simulink model "roach2_sin"  (Simulink 7.9)
settings: Solver=ode45  StartTime=0.0  StopTime=50000  RelTol=1e-3
undefined symbols (not defined in the file or in its folder): sfp
libraries: xbsIndex_r4 x2466, xps_library x13, casper_library_misc x4
(part 2 of 28)

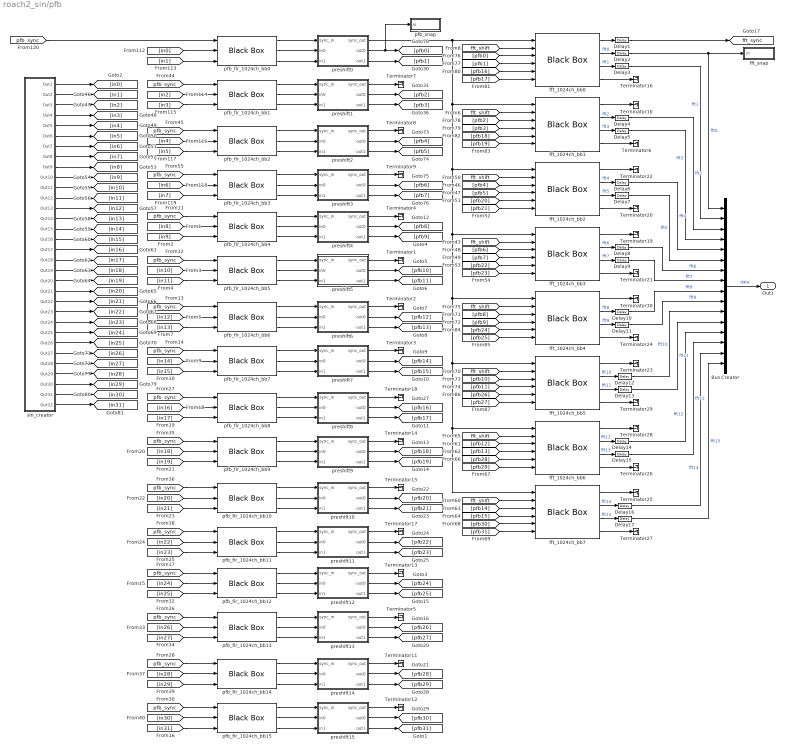
== system roach2_sin/pfb ==
  Bus Creator : BusCreator  Inputs=16
  Delay{1..17} (17 alike) : xbsIndex_r4/Delay  rst=off  infoeditControl=Selection of Reset will increase slice count due to use of real FFs and instead of SRLs  en=off  latency=1  dbl_ovrd=off  reg_retiming=off  block_type=delay
  Terminator{1..30} (30 alike) : Terminator
  fft_1024ch_bb{0..7} (8 alike) : xbsIndex_r4/Black Box  init_code=fft_1024ch_core_config  sim_method=Inactive  verbose=off  block_type=blackbox2
  fft_snap : Subsystem
  pfb_fir_1024ch_bb{0..15} (16 alike) : xbsIndex_r4/Black Box  init_code=pfb_fir_1024ch_core_config  sim_method=Inactive  verbose=off  block_type=blackbox2
  pfb_snap : Subsystem
  preshift0 : Subsystem
  preshift1 : Subsystem
  preshift10 : Subsystem
  preshift11 : Subsystem
  preshift12 : Subsystem
  preshift13 : Subsystem
  preshift14 : Subsystem
  preshift15 : Subsystem
  preshift2 : Subsystem
  preshift3 : Subsystem
  preshift4 : Subsystem
  preshift5 : Subsystem
  preshift6 : Subsystem
  preshift7 : Subsystem
  preshift8 : Subsystem
  preshift9 : Subsystem
  sin_creator : Subsystem
  Out1 : Outport
  tag scope: fft_sync=global
  wires:
  [in0] -> pfb_fir_1024ch_bb0/2
  [in1] -> pfb_fir_1024ch_bb0/3
  [in2] -> pfb_fir_1024ch_bb1/2
  [in3] -> pfb_fir_1024ch_bb1/3
  [in4] -> pfb_fir_1024ch_bb2/2
  [in5] -> pfb_fir_1024ch_bb2/3
  [in6] -> pfb_fir_1024ch_bb3/2
  [in7] -> pfb_fir_1024ch_bb3/3
  pfb_fir_1024ch_bb1/2 -> preshift1/2(in0)
  pfb_fir_1024ch_bb1/3 -> preshift1/3(in1)
  pfb_fir_1024ch_bb2/2 -> preshift2/2(in0)
  pfb_fir_1024ch_bb2/3 -> preshift2/3(in1)
  pfb_fir_1024ch_bb3/2 -> preshift3/2(in0)
  pfb_fir_1024ch_bb3/3 -> preshift3/3(in1)
  [fft_shift] -> fft_1024ch_bb1/2
  fft_1024ch_bb1/4 -> Terminator6/1
  fft_1024ch_bb0/4 -> Terminator16/1
  Delay1/1 -> [fft_sync]
  fft_1024ch_bb1/1 -> Terminator10/1
  [fft_shift] -> fft_1024ch_bb0/2
  [pfb_sync] -> pfb_fir_1024ch_bb0/1
  [pfb_sync] -> pfb_fir_1024ch_bb1/1
  [pfb_sync] -> pfb_fir_1024ch_bb2/1
  [pfb_sync] -> pfb_fir_1024ch_bb3/1
  pfb_fir_1024ch_bb1/1 -> preshift1/1(sync_in)
  pfb_fir_1024ch_bb2/1 -> preshift2/1(sync_in)
  pfb_fir_1024ch_bb3/1 -> preshift3/1(sync_in)
  pfb_fir_1024ch_bb0/1 -> preshift0/1(sync_in)
  pfb_fir_1024ch_bb0/2 -> preshift0/2(in0)
  pfb_fir_1024ch_bb0/3 -> preshift0/3(in1)
  preshift0/1(sync_out) -> fft_1024ch_bb6/1, fft_1024ch_bb7/1, fft_1024ch_bb5/1, fft_1024ch_bb4/1, fft_1024ch_bb3/1, fft_1024ch_bb2/1, fft_1024ch_bb1/1, fft_1024ch_bb0/1
  preshift0/2(out0) -> [pfb0], pfb_snap/1(in)
  preshift0/3(out1) -> [pfb1]
  preshift1/1(sync_out) -> Terminator7/1
  preshift2/1(sync_out) -> Terminator8/1
  preshift3/1(sync_out) -> Terminator9/1
  preshift1/2(out0) -> [pfb2]
  preshift1/3(out1) -> [pfb3]
  preshift2/2(out0) -> [pfb4]
  preshift2/3(out1) -> [pfb5]
  preshift3/2(out0) -> [pfb6]
  preshift3/3(out1) -> [pfb7]
  [pfb0] -> fft_1024ch_bb0/3
  [pfb1] -> fft_1024ch_bb0/4
  [pfb16] -> fft_1024ch_bb0/5
  [pfb17] -> fft_1024ch_bb0/6
  [pfb2] -> fft_1024ch_bb1/3
  [pfb3] -> fft_1024ch_bb1/4
  [pfb18] -> fft_1024ch_bb1/5
  [pfb19] -> fft_1024ch_bb1/6
  [in8] -> pfb_fir_1024ch_bb4/2
  [in9] -> pfb_fir_1024ch_bb4/3
  [in10] -> pfb_fir_1024ch_bb5/2
  [in11] -> pfb_fir_1024ch_bb5/3
  [in12] -> pfb_fir_1024ch_bb6/2
  [in13] -> pfb_fir_1024ch_bb6/3
  [in14] -> pfb_fir_1024ch_bb7/2
  [in15] -> pfb_fir_1024ch_bb7/3
  pfb_fir_1024ch_bb5/2 -> preshift5/2(in0)
  pfb_fir_1024ch_bb5/3 -> preshift5/3(in1)
  pfb_fir_1024ch_bb6/2 -> preshift6/2(in0)
  pfb_fir_1024ch_bb6/3 -> preshift6/3(in1)
  pfb_fir_1024ch_bb7/2 -> preshift7/2(in0)
  pfb_fir_1024ch_bb7/3 -> preshift7/3(in1)
  [pfb_sync] -> pfb_fir_1024ch_bb4/1
  [pfb_sync] -> pfb_fir_1024ch_bb5/1
  [pfb_sync] -> pfb_fir_1024ch_bb6/1
  [pfb_sync] -> pfb_fir_1024ch_bb7/1
  pfb_fir_1024ch_bb5/1 -> preshift5/1(sync_in)
  pfb_fir_1024ch_bb6/1 -> preshift6/1(sync_in)
  pfb_fir_1024ch_bb7/1 -> preshift7/1(sync_in)
  pfb_fir_1024ch_bb4/1 -> preshift4/1(sync_in)
  pfb_fir_1024ch_bb4/2 -> preshift4/2(in0)
  pfb_fir_1024ch_bb4/3 -> preshift4/3(in1)
  preshift4/1(sync_out) -> Terminator4/1
  preshift4/2(out0) -> [pfb8]
  preshift4/3(out1) -> [pfb9]
  preshift5/1(sync_out) -> Terminator1/1
  preshift6/1(sync_out) -> Terminator2/1
  preshift7/1(sync_out) -> Terminator3/1
  preshift5/2(out0) -> [pfb10]
  preshift5/3(out1) -> [pfb11]
  preshift6/2(out0) -> [pfb12]
  preshift6/3(out1) -> [pfb13]
  preshift7/2(out0) -> [pfb14]
  preshift7/3(out1) -> [pfb15]
  [in16] -> pfb_fir_1024ch_bb8/2
  [in17] -> pfb_fir_1024ch_bb8/3
  [in18] -> pfb_fir_1024ch_bb9/2
  [in19] -> pfb_fir_1024ch_bb9/3
  [in20] -> pfb_fir_1024ch_bb10/2
  [in21] -> pfb_fir_1024ch_bb10/3
  [in22] -> pfb_fir_1024ch_bb11/2
  [in23] -> pfb_fir_1024ch_bb11/3
  pfb_fir_1024ch_bb9/2 -> preshift9/2(in0)
  pfb_fir_1024ch_bb9/3 -> preshift9/3(in1)
  pfb_fir_1024ch_bb10/2 -> preshift10/2(in0)
  pfb_fir_1024ch_bb10/3 -> preshift10/3(in1)
  pfb_fir_1024ch_bb11/2 -> preshift11/2(in0)
  pfb_fir_1024ch_bb11/3 -> preshift11/3(in1)
  [pfb_sync] -> pfb_fir_1024ch_bb8/1
  [pfb_sync] -> pfb_fir_1024ch_bb9/1
  [pfb_sync] -> pfb_fir_1024ch_bb10/1
  [pfb_sync] -> pfb_fir_1024ch_bb11/1
  pfb_fir_1024ch_bb9/1 -> preshift9/1(sync_in)
  pfb_fir_1024ch_bb10/1 -> preshift10/1(sync_in)
  pfb_fir_1024ch_bb11/1 -> preshift11/1(sync_in)
  pfb_fir_1024ch_bb8/1 -> preshift8/1(sync_in)
  pfb_fir_1024ch_bb8/2 -> preshift8/2(in0)
  pfb_fir_1024ch_bb8/3 -> preshift8/3(in1)
  preshift8/1(sync_out) -> Terminator18/1
  preshift8/2(out0) -> [pfb16]
  preshift8/3(out1) -> [pfb17]
  preshift9/1(sync_out) -> Terminator14/1
  preshift10/1(sync_out) -> Terminator15/1
  preshift11/1(sync_out) -> Terminator17/1
  preshift9/2(out0) -> [pfb18]
  preshift9/3(out1) -> [pfb19]
  preshift10/2(out0) -> [pfb20]
  preshift10/3(out1) -> [pfb21]
  preshift11/2(out0) -> [pfb22]
  preshift11/3(out1) -> [pfb23]
  [in24] -> pfb_fir_1024ch_bb12/2
  [in25] -> pfb_fir_1024ch_bb12/3
  [in26] -> pfb_fir_1024ch_bb13/2
  [in27] -> pfb_fir_1024ch_bb13/3
  [in28] -> pfb_fir_1024ch_bb14/2
  [in29] -> pfb_fir_1024ch_bb14/3
  [in30] -> pfb_fir_1024ch_bb15/2
  [in31] -> pfb_fir_1024ch_bb15/3
  pfb_fir_1024ch_bb13/2 -> preshift13/2(in0)
  pfb_fir_1024ch_bb13/3 -> preshift13/3(in1)
  pfb_fir_1024ch_bb14/2 -> preshift14/2(in0)
  pfb_fir_1024ch_bb14/3 -> preshift14/3(in1)
  pfb_fir_1024ch_bb15/2 -> preshift15/2(in0)
  pfb_fir_1024ch_bb15/3 -> preshift15/3(in1)
  [pfb_sync] -> pfb_fir_1024ch_bb12/1
  [pfb_sync] -> pfb_fir_1024ch_bb13/1
  [pfb_sync] -> pfb_fir_1024ch_bb14/1
  [pfb_sync] -> pfb_fir_1024ch_bb15/1
  pfb_fir_1024ch_bb13/1 -> preshift13/1(sync_in)
  pfb_fir_1024ch_bb14/1 -> preshift14/1(sync_in)
  pfb_fir_1024ch_bb15/1 -> preshift15/1(sync_in)
  pfb_fir_1024ch_bb12/1 -> preshift12/1(sync_in)
  pfb_fir_1024ch_bb12/2 -> preshift12/2(in0)
  pfb_fir_1024ch_bb12/3 -> preshift12/3(in1)
  preshift12/1(sync_out) -> Terminator13/1
  preshift12/2(out0) -> [pfb24]
  preshift12/3(out1) -> [pfb25]
  preshift13/1(sync_out) -> Terminator5/1
  preshift14/1(sync_out) -> Terminator11/1
  preshift15/1(sync_out) -> Terminator12/1
  preshift13/2(out0) -> [pfb26]
  preshift13/3(out1) -> [pfb27]
  preshift14/2(out0) -> [pfb28]
  preshift14/3(out1) -> [pfb29]
  preshift15/2(out0) -> [pfb30]
  preshift15/3(out1) -> [pfb31]
  [fft_shift] -> fft_1024ch_bb3/2
  fft_1024ch_bb3/4 -> Terminator21/1
  fft_1024ch_bb2/4 -> Terminator20/1
  fft_1024ch_bb2/1 -> Terminator22/1
  fft_1024ch_bb3/1 -> Terminator19/1
  [fft_shift] -> fft_1024ch_bb2/2
  [pfb4] -> fft_1024ch_bb2/3
  [pfb5] -> fft_1024ch_bb2/4
  [pfb20] -> fft_1024ch_bb2/5
  [pfb21] -> fft_1024ch_bb2/6
  [pfb6] -> fft_1024ch_bb3/3
  [pfb7] -> fft_1024ch_bb3/4
  [pfb22] -> fft_1024ch_bb3/5
  [pfb23] -> fft_1024ch_bb3/6
  [fft_shift] -> fft_1024ch_bb5/2
  fft_1024ch_bb5/4 -> Terminator29/1
  fft_1024ch_bb4/4 -> Terminator24/1
  fft_1024ch_bb4/1 -> Terminator30/1
  fft_1024ch_bb5/1 -> Terminator23/1
  [fft_shift] -> fft_1024ch_bb4/2
  [pfb8] -> fft_1024ch_bb4/3
  [pfb9] -> fft_1024ch_bb4/4
  [pfb24] -> fft_1024ch_bb4/5
  [pfb25] -> fft_1024ch_bb4/6
  [pfb10] -> fft_1024ch_bb5/3
  [pfb11] -> fft_1024ch_bb5/4
  [pfb26] -> fft_1024ch_bb5/5
  [pfb27] -> fft_1024ch_bb5/6
  [fft_shift] -> fft_1024ch_bb7/2
  fft_1024ch_bb7/4 -> Terminator27/1
  fft_1024ch_bb6/4 -> Terminator26/1
  fft_1024ch_bb6/1 -> Terminator28/1
  fft_1024ch_bb7/1 -> Terminator25/1
  [fft_shift] -> fft_1024ch_bb6/2
  [pfb12] -> fft_1024ch_bb6/3
  [pfb13] -> fft_1024ch_bb6/4
  [pfb28] -> fft_1024ch_bb6/5
  [pfb29] -> fft_1024ch_bb6/6
  [pfb14] -> fft_1024ch_bb7/3
  [pfb15] -> fft_1024ch_bb7/4
  [pfb30] -> fft_1024ch_bb7/5
  [pfb31] -> fft_1024ch_bb7/6
  Delay2/1 -> Bus Creator/1, fft_snap/1(in)  "fft0"
  Delay3/1 -> Bus Creator/2  "fft1"
  Delay4/1 -> Bus Creator/3  "fft2"
  Delay5/1 -> Bus Creator/4  "fft3"
  Delay6/1 -> Bus Creator/5  "fft4"
  Delay7/1 -> Bus Creator/6  "fft5"
  Delay8/1 -> Bus Creator/7  "fft6"
  Delay9/1 -> Bus Creator/8  "fft7"
  Delay10/1 -> Bus Creator/9  "fft8"
  Delay11/1 -> Bus Creator/10  "fft9"
  Delay12/1 -> Bus Creator/11  "fft10"
  Delay13/1 -> Bus Creator/12  "fft11"
  Delay14/1 -> Bus Creator/13  "fft12"
  Delay15/1 -> Bus Creator/14  "fft13"
  Delay16/1 -> Bus Creator/15  "fft14"
  Delay17/1 -> Bus Creator/16  "fft15"
  Bus Creator/1 -> Out1/1  "data"
  fft_1024ch_bb7/2 -> Delay16/1  "fft14"
  fft_1024ch_bb7/3 -> Delay17/1  "fft15"
  fft_1024ch_bb6/2 -> Delay14/1  "fft12"
  fft_1024ch_bb6/3 -> Delay15/1  "fft13"
  fft_1024ch_bb5/2 -> Delay12/1  "fft10"
  fft_1024ch_bb5/3 -> Delay13/1  "fft11"
  fft_1024ch_bb0/2 -> Delay2/1  "fft0"
  fft_1024ch_bb0/1 -> Delay1/1
  fft_1024ch_bb0/3 -> Delay3/1  "fft1"
  fft_1024ch_bb1/2 -> Delay4/1  "fft2"
  fft_1024ch_bb1/3 -> Delay5/1  "fft3"
  fft_1024ch_bb2/2 -> Delay6/1  "fft4"
  fft_1024ch_bb2/3 -> Delay7/1  "fft5"
  fft_1024ch_bb3/2 -> Delay8/1  "fft6"
  fft_1024ch_bb3/3 -> Delay9/1  "fft7"
  fft_1024ch_bb4/2 -> Delay10/1  "fft8"
  fft_1024ch_bb4/3 -> Delay11/1  "fft9"
  sin_creator/1(Out1) -> [in0]
  sin_creator/2(Out2) -> [in1]
  sin_creator/3(Out3) -> [in2]
  sin_creator/4(Out4) -> [in3]
  sin_creator/5(Out5) -> [in4]
  sin_creator/6(Out6) -> [in5]
  sin_creator/7(Out7) -> [in6]
  sin_creator/8(Out8) -> [in7]
  sin_creator/9(Out9) -> [in8]
  sin_creator/10(Out10) -> [in9]
  sin_creator/11(Out11) -> [in10]
  sin_creator/12(Out12) -> [in11]
  sin_creator/13(Out13) -> [in12]
  sin_creator/14(Out14) -> [in13]
  sin_creator/15(Out15) -> [in14]
  sin_creator/16(Out16) -> [in15]
  sin_creator/17(Out17) -> [in16]
  sin_creator/18(Out18) -> [in17]
  sin_creator/19(Out19) -> [in18]
  sin_creator/20(Out20) -> [in19]
  sin_creator/21(Out21) -> [in20]
  sin_creator/22(Out22) -> [in21]
  sin_creator/23(Out23) -> [in22]
  sin_creator/24(Out24) -> [in23]
  sin_creator/25(Out25) -> [in24]
  sin_creator/26(Out26) -> [in25]
  sin_creator/27(Out27) -> [in26]
  sin_creator/28(Out28) -> [in27]
  sin_creator/29(Out29) -> [in28]
  sin_creator/30(Out30) -> [in29]
  sin_creator/31(Out31) -> [in30]
  sin_creator/32(Out32) -> [in31]

== system roach2_sin/rcs ==
  note: 0 -> revision info
      | 1 -> timestamp
  note: Last modification
      | in seconds since
      | 01-01-1970
  note: 0 -> git
      | 1 -> svn
  note: 0 -> cleanly in revision control
  note: git -> first 28 bits of commit hash
      | svn -> latest revision number
  note: 0 -> revision info
      | 1 -> timestamp
  note: Compile time
      | in seconds since
      | 01-01-1970
  note: 0 -> git
      | 1 -> svn
  note: 0 -> cleanly in revision control
  note: git -> first 28 bits of commit hash
      | svn -> latest revision number
  Concat : xbsIndex_r4/Concat  num_inputs=2  dbl_ovrd=off  block_type=concat
  Concat1 : xbsIndex_r4/Concat  num_inputs=2  dbl_ovrd=off  block_type=concat
  Mux : xbsIndex_r4/Mux  inputs=2  en=off  latency=0  precision=Full  arith_type=Unsigned  n_bits=16  bin_pt=14  quantization=Truncate  overflow=Wrap  dbl_ovrd=off  block_type=mux
      settings: block_version=11.4
  Mux1 : xbsIndex_r4/Mux  inputs=2  en=off  latency=0  precision=Full  arith_type=Unsigned  n_bits=16  bin_pt=14  quantization=Truncate  overflow=Wrap  dbl_ovrd=off  block_type=mux
      settings: block_version=11.4
  Terminator : Terminator
  Terminator1 : Terminator
  Terminator2 : Terminator
  app : Subsystem  Tag=xps:sw_reg  mask{io_dir=To Processor, arith_type=Unsigned, bitwidth=32, bin_pt=0, sample_period=1, latency=0}
      mask prompts: io_dir "I/O direction"; arith_type "Data Type"; bitwidth "Data bitwidth"; bin_pt "Data binary point"; sample_period "Sample period"
      mask init:
      | gw_name = clear_name(gcb);
      | config.source = str2func('software_register_init');
      | config.toplevel = gcb;
      | xBlock( config, {io_dir, arith_type, bitwidth, bin_pt, ... 
      |     sample_period, gw_name, latency});
      mask StyleString: popup(From Processor|To Processor),popup(Unsigned|Signed  (2's comp)),edit,edit,edit,edit
  app_dirty : xbsIndex_r4/Constant  const=1  gui_display_data_type=Fixed-point  arith_type=Unsigned  n_bits=1  bin_pt=0  preci_type=Single  exp_width=8  frac_width=24  explicit_period=on  period=1  equ=P=C  opselect=C  inp2=PCIN>>17  opr=+  inp1=P  carry=CIN  dbl_ovrd=off  block_type=constant
      settings: block_version=11.4
  app_info : xbsIndex_r4/Concat  num_inputs=4  dbl_ovrd=off  block_type=concat
  app_info1 : xbsIndex_r4/Concat  num_inputs=4  dbl_ovrd=off  block_type=concat
  app_rcs_type : xbsIndex_r4/Constant  const=0  gui_display_data_type=Fixed-point  arith_type=Unsigned  n_bits=1  bin_pt=0  preci_type=Single  exp_width=8  frac_width=24  explicit_period=on  period=1  equ=P=C  opselect=C  inp2=PCIN>>17  opr=+  inp1=P  carry=CIN  dbl_ovrd=off  block_type=constant
      settings: block_version=11.4
  app_reserved : xbsIndex_r4/Constant  const=0  gui_display_data_type=Fixed-point  arith_type=Unsigned  n_bits=1  bin_pt=0  preci_type=Single  exp_width=8  frac_width=24  explicit_period=on  period=1  equ=P=C  opselect=C  inp2=PCIN>>17  opr=+  inp1=P  carry=CIN  dbl_ovrd=off  block_type=constant
      settings: block_version=11.4
  app_revision : xbsIndex_r4/Constant  const=0  gui_display_data_type=Fixed-point  arith_type=Unsigned  n_bits=28  bin_pt=0  preci_type=Single  exp_width=8  frac_width=24  explicit_period=on  period=1  equ=P=C  opselect=C  inp2=PCIN>>17  opr=+  inp1=P  carry=CIN  dbl_ovrd=off  block_type=constant
      settings: block_version=11.4
  app_timestamp : xbsIndex_r4/Constant  const=1445445588  gui_display_data_type=Fixed-point  arith_type=Unsigned  n_bits=31  bin_pt=0  preci_type=Single  exp_width=8  frac_width=24  explicit_period=on  period=1  equ=P=C  opselect=C  inp2=PCIN>>17  opr=+  inp1=P  carry=CIN  dbl_ovrd=off  block_type=constant
      settings: block_version=11.4
  app_type : xbsIndex_r4/Constant  const=1  gui_display_data_type=Fixed-point  arith_type=Unsigned  n_bits=1  bin_pt=0  preci_type=Single  exp_width=8  frac_width=24  explicit_period=on  period=1  equ=P=C  opselect=C  inp2=PCIN>>17  opr=+  inp1=P  carry=CIN  dbl_ovrd=off  block_type=constant
      settings: block_version=11.4
  lib : Subsystem  Tag=xps:sw_reg  mask{io_dir=To Processor, arith_type=Unsigned, bitwidth=32, bin_pt=0, sample_period=1, latency=0}
      mask prompts: io_dir "I/O direction"; arith_type "Data Type"; bitwidth "Data bitwidth"; bin_pt "Data binary point"; sample_period "Sample period"
      mask init:
      | gw_name = clear_name(gcb);
      | config.source = str2func('software_register_init');
      | config.toplevel = gcb;
      | xBlock( config, {io_dir, arith_type, bitwidth, bin_pt, ... 
      |     sample_period, gw_name, latency});
      mask StyleString: popup(From Processor|To Processor),popup(Unsigned|Signed  (2's comp)),edit,edit,edit,edit
  lib_dirty : xbsIndex_r4/Constant  const=1  gui_display_data_type=Fixed-point  arith_type=Unsigned  n_bits=1  bin_pt=0  preci_type=Single  exp_width=8  frac_width=24  explicit_period=on  period=1  equ=P=C  opselect=C  inp2=PCIN>>17  opr=+  inp1=P  carry=CIN  dbl_ovrd=off  block_type=constant
      settings: block_version=11.4
  lib_rcs_type : xbsIndex_r4/Constant  const=0  gui_display_data_type=Fixed-point  arith_type=Unsigned  n_bits=1  bin_pt=0  preci_type=Single  exp_width=8  frac_width=24  explicit_period=on  period=1  equ=P=C  opselect=C  inp2=PCIN>>17  opr=+  inp1=P  carry=CIN  dbl_ovrd=off  block_type=constant
      settings: block_version=11.4
  lib_reserved : xbsIndex_r4/Constant  const=0  gui_display_data_type=Fixed-point  arith_type=Unsigned  n_bits=1  bin_pt=0  preci_type=Single  exp_width=8  frac_width=24  explicit_period=on  period=1  equ=P=C  opselect=C  inp2=PCIN>>17  opr=+  inp1=P  carry=CIN  dbl_ovrd=off  block_type=constant
      settings: block_version=11.4
  lib_revision : xbsIndex_r4/Constant  const=hex2dec('2f40dad')  gui_display_data_type=Fixed-point  arith_type=Unsigned  n_bits=28  bin_pt=0  preci_type=Single  exp_width=8  frac_width=24  explicit_period=on  period=1  equ=P=C  opselect=C  inp2=PCIN>>17  opr=+  inp1=P  carry=CIN  dbl_ovrd=off  block_type=constant
      settings: block_version=11.4
  lib_timestamp : xbsIndex_r4/Constant  const=1445474578  gui_display_data_type=Fixed-point  arith_type=Unsigned  n_bits=31  bin_pt=0  preci_type=Single  exp_width=8  frac_width=24  explicit_period=on  period=1  equ=P=C  opselect=C  inp2=PCIN>>17  opr=+  inp1=P  carry=CIN  dbl_ovrd=off  block_type=constant
      settings: block_version=11.4
  lib_type : xbsIndex_r4/Constant  const=0  gui_display_data_type=Fixed-point  arith_type=Unsigned  n_bits=1  bin_pt=0  preci_type=Single  exp_width=8  frac_width=24  explicit_period=on  period=1  equ=P=C  opselect=C  inp2=PCIN>>17  opr=+  inp1=P  carry=CIN  dbl_ovrd=off  block_type=constant
      settings: block_version=11.4
  user : Subsystem  Tag=xps:sw_reg  mask{io_dir=To Processor, arith_type=Unsigned, bitwidth=32, bin_pt=0, sample_period=1, latency=0}
      mask prompts: io_dir "I/O direction"; arith_type "Data Type"; bitwidth "Data bitwidth"; bin_pt "Data binary point"; sample_period "Sample period"
      mask init:
      | gw_name = clear_name(gcb);
      | config.source = str2func('software_register_init');
      | config.toplevel = gcb;
      | xBlock( config, {io_dir, arith_type, bitwidth, bin_pt, ... 
      |     sample_period, gw_name, latency});
      mask StyleString: popup(From Processor|To Processor),popup(Unsigned|Signed  (2's comp)),edit,edit,edit,edit
  user_val : xbsIndex_r4/Constant  const=user_val  gui_display_data_type=Fixed-point  arith_type=Unsigned  n_bits=32  bin_pt=0  preci_type=Single  exp_width=8  frac_width=24  explicit_period=off  period=1  equ=P=C  opselect=C  inp2=PCIN>>17  opr=+  inp1=P  carry=CIN  dbl_ovrd=off  block_type=constant
      settings: block_version=11.4
  wires:
  app/1(sim_reg_out) -> Terminator/1
  user/1(sim_reg_out) -> Terminator2/1
  user_val/1 -> user/1(reg_out)
  app_rcs_type/1 -> app_info/1
  app_reserved/1 -> app_info/2
  app_dirty/1 -> app_info/3
  app_revision/1 -> app_info/4
  app_type/1 -> Concat/1, Mux/1
  app_info/1 -> Mux/2
  app_timestamp/1 -> Mux/3
  Mux/1 -> Concat/2
  Concat/1 -> app/1(reg_out)
  lib/1(sim_reg_out) -> Terminator1/1
  lib_rcs_type/1 -> app_info1/1
  lib_reserved/1 -> app_info1/2
  lib_dirty/1 -> app_info1/3
  lib_revision/1 -> app_info1/4
  lib_type/1 -> Concat1/1, Mux1/1
  app_info1/1 -> Mux1/2
  lib_timestamp/1 -> Mux1/3
  Mux1/1 -> Concat1/2
  Concat1/1 -> lib/1(reg_out)

== system roach2_sin/seed ==
  0 : Subsystem  Tag=xps:sw_reg  AncestorBlock=xps_library/software register  mask{numios=1, io_dir=From Processor, io_delay=0, sample_period=1, name1=reg, bitwidth1=32, arith_type1=Unsigned, bin_pt1=0}
      mask prompts: numios "Num I/Os"; io_dir "I/O direction"; io_delay "I/O delay"; sample_period "Sample period"; name1 "Name"; bitwidth1 "Bitwidth"; arith_type1 "Type"; bin_pt1 "Binary pt"
      mask init:
      | swreg_init(gcb);
      mask StyleString: edit,popup(To Processor|From Processor),edit,edit,edit,edit,popup(Unsigned|Signed  (2's comp)|Boolean),edit
      mask CallbackString:
      | t = timer('StartDelay', 0.1); t.TimerFcn = {@swreg_cb, gcb}; start(t);|a = get_param(gcb, 'MaskEnables');
      | if strcmp(get_param(gcb, 'io_dir'), 'To Processor'),
      | a(4) = {'off'};
      | else
      | a(4) = {'on'};
      | end
      | set_param(gcb, 'MaskEnables', a);
      | |||||a = get_param(gcb, 'MaskEnables');
      | if strcmp(get_param(gcb, 'arith_type1'), 'Boolean'),
      | set_param(gcb, 'bitwidth1', '1');
      | set_param(gcb, 'bin_pt1', '0');
      | a(6) = {'off'};
      | a(8) = {'off'};
      | else
      | a(6) = {'on'};
      | a(8) = {'on'};
      | end
      | set_param(gcb, 'MaskEnables', a);
      | |
      mask TabNameString: Setup,Setup,Setup,Setup,IO1,IO1,IO1,IO1
  1 : Subsystem  Tag=xps:sw_reg  AncestorBlock=xps_library/software register  mask{numios=1, io_dir=From Processor, io_delay=0, sample_period=1, name1=reg, bitwidth1=32, arith_type1=Unsigned, bin_pt1=0}
      mask prompts: numios "Num I/Os"; io_dir "I/O direction"; io_delay "I/O delay"; sample_period "Sample period"; name1 "Name"; bitwidth1 "Bitwidth"; arith_type1 "Type"; bin_pt1 "Binary pt"
      mask init:
      | swreg_init(gcb);
      mask StyleString: edit,popup(To Processor|From Processor),edit,edit,edit,edit,popup(Unsigned|Signed  (2's comp)|Boolean),edit
      mask CallbackString:
      | t = timer('StartDelay', 0.1); t.TimerFcn = {@swreg_cb, gcb}; start(t);|a = get_param(gcb, 'MaskEnables');
      | if strcmp(get_param(gcb, 'io_dir'), 'To Processor'),
      | a(4) = {'off'};
      | else
      | a(4) = {'on'};
      | end
      | set_param(gcb, 'MaskEnables', a);
      | |||||a = get_param(gcb, 'MaskEnables');
      | if strcmp(get_param(gcb, 'arith_type1'), 'Boolean'),
      | set_param(gcb, 'bitwidth1', '1');
      | set_param(gcb, 'bin_pt1', '0');
      | a(6) = {'off'};
      | a(8) = {'off'};
      | else
      | a(6) = {'on'};
      | a(8) = {'on'};
      | end
      | set_param(gcb, 'MaskEnables', a);
      | |
      mask TabNameString: Setup,Setup,Setup,Setup,IO1,IO1,IO1,IO1
  2 : Subsystem  Tag=xps:sw_reg  AncestorBlock=xps_library/software register  mask{numios=1, io_dir=From Processor, io_delay=0, sample_period=1, name1=reg, bitwidth1=32, arith_type1=Unsigned, bin_pt1=0}
      mask prompts: numios "Num I/Os"; io_dir "I/O direction"; io_delay "I/O delay"; sample_period "Sample period"; name1 "Name"; bitwidth1 "Bitwidth"; arith_type1 "Type"; bin_pt1 "Binary pt"
      mask init:
      | swreg_init(gcb);
      mask StyleString: edit,popup(To Processor|From Processor),edit,edit,edit,edit,popup(Unsigned|Signed  (2's comp)|Boolean),edit
      mask CallbackString:
      | t = timer('StartDelay', 0.1); t.TimerFcn = {@swreg_cb, gcb}; start(t);|a = get_param(gcb, 'MaskEnables');
      | if strcmp(get_param(gcb, 'io_dir'), 'To Processor'),
      | a(4) = {'off'};
      | else
      | a(4) = {'on'};
      | end
      | set_param(gcb, 'MaskEnables', a);
      | |||||a = get_param(gcb, 'MaskEnables');
      | if strcmp(get_param(gcb, 'arith_type1'), 'Boolean'),
      | set_param(gcb, 'bitwidth1', '1');
      | set_param(gcb, 'bin_pt1', '0');
      | a(6) = {'off'};
      | a(8) = {'off'};
      | else
      | a(6) = {'on'};
      | a(8) = {'on'};
      | end
      | set_param(gcb, 'MaskEnables', a);
      | |
      mask TabNameString: Setup,Setup,Setup,Setup,IO1,IO1,IO1,IO1
  3 : Subsystem  Tag=xps:sw_reg  AncestorBlock=xps_library/software register  mask{numios=1, io_dir=From Processor, io_delay=0, sample_period=1, name1=reg, bitwidth1=32, arith_type1=Unsigned, bin_pt1=0}
      mask prompts: numios "Num I/Os"; io_dir "I/O direction"; io_delay "I/O delay"; sample_period "Sample period"; name1 "Name"; bitwidth1 "Bitwidth"; arith_type1 "Type"; bin_pt1 "Binary pt"
      mask init:
      | swreg_init(gcb);
      mask StyleString: edit,popup(To Processor|From Processor),edit,edit,edit,edit,popup(Unsigned|Signed  (2's comp)|Boolean),edit
      mask CallbackString:
      | t = timer('StartDelay', 0.1); t.TimerFcn = {@swreg_cb, gcb}; start(t);|a = get_param(gcb, 'MaskEnables');
      | if strcmp(get_param(gcb, 'io_dir'), 'To Processor'),
      | a(4) = {'off'};
      | else
      | a(4) = {'on'};
      | end
      | set_param(gcb, 'MaskEnables', a);
      | |||||a = get_param(gcb, 'MaskEnables');
      | if strcmp(get_param(gcb, 'arith_type1'), 'Boolean'),
      | set_param(gcb, 'bitwidth1', '1');
      | set_param(gcb, 'bin_pt1', '0');
      | a(6) = {'off'};
      | a(8) = {'off'};
      | else
      | a(6) = {'on'};
      | a(8) = {'on'};
      | end
      | set_param(gcb, 'MaskEnables', a);
      | |
      mask TabNameString: Setup,Setup,Setup,Setup,IO1,IO1,IO1,IO1
  BitBasher : xbsIndex_r4/BitBasher  bitexpr=|  display_expr=off  sr_1=1  arith_type1=Unsigned  bin_pt1=0  sr_2=2  arith_type2=Unsigned  bin_pt2=0  sr_3=3  arith_type3=Unsigned  bin_pt3=0  sr_4=4  arith_type4=Unsigned  bin_pt4=0  dbl_ovrd=off  block_type=bitbasher
      | <!DOCTYPE HTML PUBLIC "-//W3C//DTD HTML 4.0//EN" "http://www.w3.org/TR/REC-html40/strict.dtd">
      | <html><head><meta name="qrichtext" content="1" /><style type="text/css">
      | p, li { white-space: pre-wrap; }
      | </style></head><body style=" font-family:'Sans'; font-size:10pt; font-weight:400; font-style:normal;">
      | <p style=" margin-top:12px; margin-bottom:12px; margin-left:0px; margin-right:0px; -qt-block-indent:0; text-indent:0px;"><span style=" font-family:'MS Shell Dlg'; font-size:8pt;">s0={8'h5c, s32[7:0], s32[0:7], 8'ha3}<br />s1={8'h5c, s32[15:8], s32[8:15], 8'ha3}<br />s2={8'h5c, s32[23:16], s32[16:23], 8'ha3}<br />s3={8'h5c, s32[31:24], s32[24:31], 8'ha3}</span></p></body></html>
  BitBasher1 : xbsIndex_r4/BitBasher  bitexpr=|  display_expr=off  sr_1=1  arith_type1=Unsigned  bin_pt1=0  sr_2=2  arith_type2=Unsigned  bin_pt2=0  sr_3=3  arith_type3=Unsigned  bin_pt3=0  sr_4=4  arith_type4=Unsigned  bin_pt4=0  dbl_ovrd=off  block_type=bitbasher
      | <!DOCTYPE HTML PUBLIC "-//W3C//DTD HTML 4.0//EN" "http://www.w3.org/TR/REC-html40/strict.dtd">
      | <html><head><meta name="qrichtext" content="1" /><style type="text/css">
      | p, li { white-space: pre-wrap; }
      | </style></head><body style=" font-family:'Sans'; font-size:10pt; font-weight:400; font-style:normal;">
      | <p style=" margin-top:12px; margin-bottom:12px; margin-left:0px; margin-right:0px; -qt-block-indent:0; text-indent:0px;"><span style=" font-family:'MS Shell Dlg'; font-size:8pt;">s0={8'h5c, s32[7:0], s32[0:7], 8'ha3}<br />s1={8'h5c, s32[15:8], s32[8:15], 8'ha3}<br />s2={8'h5c, s32[23:16], s32[16:23], 8'ha3}<br />s3={8'h5c, s32[31:24], s32[24:31], 8'ha3}</span></p></body></html>
  BitBasher2 : xbsIndex_r4/BitBasher  bitexpr=|  display_expr=off  sr_1=1  arith_type1=Unsigned  bin_pt1=0  sr_2=2  arith_type2=Unsigned  bin_pt2=0  sr_3=3  arith_type3=Unsigned  bin_pt3=0  sr_4=4  arith_type4=Unsigned  bin_pt4=0  dbl_ovrd=off  block_type=bitbasher
      | <!DOCTYPE HTML PUBLIC "-//W3C//DTD HTML 4.0//EN" "http://www.w3.org/TR/REC-html40/strict.dtd">
      | <html><head><meta name="qrichtext" content="1" /><style type="text/css">
      | p, li { white-space: pre-wrap; }
      | </style></head><body style=" font-family:'Sans'; font-size:10pt; font-weight:400; font-style:normal;">
      | <p style=" margin-top:12px; margin-bottom:12px; margin-left:0px; margin-right:0px; -qt-block-indent:0; text-indent:0px;"><span style=" font-family:'MS Shell Dlg'; font-size:8pt;">s0={8'h5c, s32[7:0], s32[0:7], 8'ha3}<br />s1={8'h5c, s32[15:8], s32[8:15], 8'ha3}<br />s2={8'h5c, s32[23:16], s32[16:23], 8'ha3}<br />s3={8'h5c, s32[31:24], s32[24:31], 8'ha3}</span></p></body></html>
  BitBasher3 : xbsIndex_r4/BitBasher  bitexpr=|  display_expr=off  sr_1=1  arith_type1=Unsigned  bin_pt1=0  sr_2=2  arith_type2=Unsigned  bin_pt2=0  sr_3=3  arith_type3=Unsigned  bin_pt3=0  sr_4=4  arith_type4=Unsigned  bin_pt4=0  dbl_ovrd=off  block_type=bitbasher
      | <!DOCTYPE HTML PUBLIC "-//W3C//DTD HTML 4.0//EN" "http://www.w3.org/TR/REC-html40/strict.dtd">
      | <html><head><meta name="qrichtext" content="1" /><style type="text/css">
      | p, li { white-space: pre-wrap; }
      | </style></head><body style=" font-family:'Sans'; font-size:10pt; font-weight:400; font-style:normal;">
      | <p style=" margin-top:12px; margin-bottom:12px; margin-left:0px; margin-right:0px; -qt-block-indent:0; text-indent:0px;"><span style=" font-family:'MS Shell Dlg'; font-size:8pt;">s0={8'h5c, s32[7:0], s32[0:7], 8'ha3}<br />s1={8'h5c, s32[15:8], s32[8:15], 8'ha3}<br />s2={8'h5c, s32[23:16], s32[16:23], 8'ha3}<br />s3={8'h5c, s32[31:24], s32[24:31], 8'ha3}</span></p></body></html>
  Constant{,1..3} (4 alike) : Constant  Value=0
  armed_trigger : casper_library_misc/armed_trigger
  tag scope: seed0=global, seed10=global, seed11=global, seed12=global, seed13=global, seed14=global, seed15=global, seed1=global, seed2=global, seed3=global, seed4=global, seed5=global, seed6=global, seed7=global, seed8=global, seed9=global, seed_ld=global
  wires:
  Constant3/1 -> 3/1(sim_reg)
  Constant2/1 -> 2/1(sim_reg)
  Constant1/1 -> 1/1(sim_reg)
  1/1(in_reg) -> BitBasher1/1
  Constant/1 -> 0/1(sim_reg)
  0/1(in_reg) -> BitBasher/1
  BitBasher/1 -> [seed0]
  BitBasher/2 -> [seed1]
  BitBasher/3 -> [seed2]
  BitBasher/4 -> [seed3]
  BitBasher1/1 -> [seed4]
  BitBasher1/2 -> [seed5]
  BitBasher1/3 -> [seed6]
  BitBasher1/4 -> [seed7]
  BitBasher2/1 -> [seed8]
  BitBasher2/2 -> [seed9]
  BitBasher2/3 -> [seed10]
  BitBasher2/4 -> [seed11]
  BitBasher3/1 -> [seed12]
  BitBasher3/2 -> [seed13]
  BitBasher3/3 -> [seed14]
  BitBasher3/4 -> [seed15]
  3/1(in_reg) -> BitBasher3/1
  2/1(in_reg) -> BitBasher2/1
  [arm_noise] -> armed_trigger/1
  [sync_in] -> armed_trigger/2
  armed_trigger/1 -> [seed_ld]

== system roach2_sin/snap ==
  0 : Subsystem  Tag=casper:snapshot  AncestorBlock=casper_library_scopes/snapshot  mask(snapshot){storage=bram, dram_dimm=1, dram_clock=200, nsamples=12, data_width=32, offset=off, circap=off, value=off, use_dsp48=off}
      mask prompts: storage "Storage medium"; dram_dimm "DRAM dimm"; dram_clock "DRAM clock rate"; nsamples "Number of Samples (2^?)"; data_width "Data width"; offset "Start delay support"; circap "Circular capture support"; value "Extra value capture support"; use_dsp48 "Use DSP48s to implement counters"
      mask help:
      | Standard snap block, but with the following optional extras;
      |  1) Optionally delay the fabric trigger by a user-specified number of valids.
      |  2) Capture continuously after a trigger until a stop pulse is received. Data is captured into BRAM continuously, with old data being overwritten until a stop command is received. Then stop capturing when memory is filled. This enables you to get some data before and some data after the stop pulse. Use the 'tr_en_cnt' register to determine where you are in your vector. 'Addr' register's MSb now indicates 'busy capturing'.
      |  3) Optionally capture a value on an input port to a register at the same time as the first data sample.
      |  4) Implement counters using DSP48Es to save resources.
      mask init:
      | snapshot_init(gcb, ...
      |     'storage', storage, ...
      |     'dram_dimm', dram_dimm, ...
      |     'dram_clock', dram_clock, ...
      |     'nsamples', nsamples, ...
      |     'data_width', data_width, ...
      |     'offset', offset, ...
      |     'circap', circap, ...
      |     'value', value, ...
      |     'use_dsp48', use_dsp48);
      mask StyleString: popup(bram|dram),popup(1|2|3|4),popup(200|250|266|300|333),edit,popup(8|16|32|64|128),checkbox,checkbox,checkbox,checkbox
      mask CallbackString: snapshot_callback();||||||||
  0_sel : Subsystem  Tag=xps:sw_reg  AncestorBlock=xps_library/software register  mask{numios=1, io_dir=From Processor, io_delay=0, sample_period=1, name1=reg, bitwidth1=32, arith_type1=Unsigned, bin_pt1=0}
      mask prompts: numios "Num I/Os"; io_dir "I/O direction"; io_delay "I/O delay"; sample_period "Sample period"; name1 "Name"; bitwidth1 "Bitwidth"; arith_type1 "Type"; bin_pt1 "Binary pt"
      mask init:
      | swreg_init(gcb);
      mask StyleString: edit,popup(To Processor|From Processor),edit,edit,edit,edit,popup(Unsigned|Signed  (2's comp)|Boolean),edit
      mask CallbackString:
      | t = timer('StartDelay', 0.1); t.TimerFcn = {@swreg_cb, gcb}; start(t);|a = get_param(gcb, 'MaskEnables');
      | if strcmp(get_param(gcb, 'io_dir'), 'To Processor'),
      | a(4) = {'off'};
      | else
      | a(4) = {'on'};
      | end
      | set_param(gcb, 'MaskEnables', a);
      | |||||a = get_param(gcb, 'MaskEnables');
      | if strcmp(get_param(gcb, 'arith_type1'), 'Boolean'),
      | set_param(gcb, 'bitwidth1', '1');
      | set_param(gcb, 'bin_pt1', '0');
      | a(6) = {'off'};
      | a(8) = {'off'};
      | else
      | a(6) = {'on'};
      | a(8) = {'on'};
      | end
      | set_param(gcb, 'MaskEnables', a);
      | |
      mask TabNameString: Setup,Setup,Setup,Setup,IO1,IO1,IO1,IO1
  BitBasher : xbsIndex_r4/BitBasher  bitexpr=|  display_expr=off  sr_1=1  arith_type1=Unsigned  bin_pt1=0  sr_2=2  arith_type2=Unsigned  bin_pt2=0  sr_3=3  arith_type3=Unsigned  bin_pt3=0  sr_4=4  arith_type4=Unsigned  bin_pt4=0  dbl_ovrd=off  block_type=bitbasher
      | <!DOCTYPE HTML PUBLIC "-//W3C//DTD HTML 4.0//EN" "http://www.w3.org/TR/REC-html40/strict.dtd">
      | <html><head><meta name="qrichtext" content="1" /><style type="text/css">
      | p, li { white-space: pre-wrap; }
      | </style></head><body style=" font-family:'Sans'; font-size:10pt; font-weight:400; font-style:normal;">
      | <p style=" margin-top:12px; margin-bottom:12px; margin-left:0px; margin-right:0px; -qt-block-indent:0; text-indent:0px;"><span style=" font-family:'MS Shell Dlg'; font-size:8pt;">sel={reg[1:0]}</span></p></body></html>
  Constant : Constant  Value=0
  Constant1 : xbsIndex_r4/Constant  const=1  gui_display_data_type=Fixed-point  arith_type=Signed (2's comp)  n_bits=16  bin_pt=14  preci_type=Single  exp_width=8  frac_width=24  explicit_period=off  period=1  equ=P=C  opselect=C  inp2=PCIN>>17  opr=+  inp1=P  carry=CIN  dbl_ovrd=off  block_type=constant
  Mux : xbsIndex_r4/Mux  inputs=4  en=off  latency=1  precision=Full  arith_type=Unsigned  n_bits=16  bin_pt=14  quantization=Truncate  overflow=Wrap  dbl_ovrd=off  block_type=mux
  zero : xbsIndex_r4/Constant  const=0  gui_display_data_type=Fixed-point  arith_type=Boolean  n_bits=16  bin_pt=14  preci_type=Single  exp_width=8  frac_width=24  explicit_period=on  period=1  equ=P=C  opselect=C  inp2=PCIN>>17  opr=+  inp1=P  carry=CIN  dbl_ovrd=off  block_type=constant
      settings: block_version=11.4
  wires:
  Constant/1 -> 0_sel/1(sim_reg)
  0_sel/1(in_reg) -> BitBasher/1
  Constant1/1 -> Mux/2
  [pfb_snap] -> Mux/3
  [fft_snap] -> Mux/4
  [eq_snap] -> Mux/5
  BitBasher/1 -> Mux/1
  Mux/1 -> 0/1(din)
  zero/1 -> 0/2(we), 0/3(trig)

== system roach2_sin/sync ==
  note: arm[4] = sw_sync
      | arm[1] = arm_noise
      | arm[0] = arm_sync
  BitBasher : xbsIndex_r4/BitBasher  bitexpr=|  display_expr=off  sr_1=1  arith_type1=Unsigned  bin_pt1=0  sr_2=2  arith_type2=Unsigned  bin_pt2=0  sr_3=3  arith_type3=Unsigned  bin_pt3=0  sr_4=4  arith_type4=Unsigned  bin_pt4=0  dbl_ovrd=off  block_type=bitbasher
      | <!DOCTYPE HTML PUBLIC "-//W3C//DTD HTML 4.0//EN" "http://www.w3.org/TR/REC-html40/strict.dtd">
      | <html><head><meta name="qrichtext" content="1" /><style type="text/css">
      | p, li { white-space: pre-wrap; }
      | </style></head><body style=" font-family:'Sans'; font-size:10pt; font-weight:400; font-style:normal;">
      | <p style=" margin-top:12px; margin-bottom:12px; margin-left:0px; margin-right:0px; -qt-block-indent:0; text-indent:0px;"><span style=" font-family:'MS Shell Dlg'; font-size:8pt;">sw_sync={arm[4]}<br />arm_noise={arm[1]}<br />arm_sync={arm[0]}</span></p></body></html>
  Constant : Constant  Value=0
  Constant33 : Constant  Value=0
  Convert1 : xbsIndex_r4/Convert  gui_display_data_type=Boolean  arith_type=Boolean  n_bits=32  bin_pt=0  float_type=Single  exp_bits=8  fraction_bits=24  quantization=Truncate  overflow=Wrap  en=off  latency=0  dbl_ovrd=off  pipeline=off  block_type=convert
      settings: block_version=11.4
  Convert2 : xbsIndex_r4/Convert  gui_display_data_type=Boolean  arith_type=Boolean  n_bits=32  bin_pt=0  float_type=Single  exp_bits=8  fraction_bits=24  quantization=Truncate  overflow=Wrap  en=off  latency=0  dbl_ovrd=off  pipeline=off  block_type=convert
      settings: block_version=11.4
  Convert3 : xbsIndex_r4/Convert  gui_display_data_type=Boolean  arith_type=Boolean  n_bits=32  bin_pt=0  float_type=Single  exp_bits=8  fraction_bits=24  quantization=Truncate  overflow=Wrap  en=off  latency=0  dbl_ovrd=off  pipeline=off  block_type=convert
      settings: block_version=11.4
  Register{,2..4} (4 alike) : xbsIndex_r4/Register  init=0  rst=off  en=off  dbl_ovrd=off  block_type=register
  Register1 : xbsIndex_r4/Register  init=0  rst=off  en=on  dbl_ovrd=off  block_type=register
  Terminator : Terminator
  Terminator1 : Terminator
  Terminator2 : Terminator
  arm : Subsystem  Tag=xps:sw_reg  AncestorBlock=xps_library/software register  mask{numios=1, io_dir=From Processor, io_delay=0, sample_period=1, name1=reg, bitwidth1=32, arith_type1=Unsigned, bin_pt1=0}
      mask prompts: numios "Num I/Os"; io_dir "I/O direction"; io_delay "I/O delay"; sample_period "Sample period"; name1 "Name"; bitwidth1 "Bitwidth"; arith_type1 "Type"; bin_pt1 "Binary pt"
      mask init:
      | swreg_init(gcb);
      mask StyleString: edit,popup(To Processor|From Processor),edit,edit,edit,edit,popup(Unsigned|Signed  (2's comp)|Boolean),edit
      mask CallbackString:
      | t = timer('StartDelay', 0.1); t.TimerFcn = {@swreg_cb, gcb}; start(t);|a = get_param(gcb, 'MaskEnables');
      | if strcmp(get_param(gcb, 'io_dir'), 'To Processor'),
      | a(4) = {'off'};
      | else
      | a(4) = {'on'};
      | end
      | set_param(gcb, 'MaskEnables', a);
      | |||||a = get_param(gcb, 'MaskEnables');
      | if strcmp(get_param(gcb, 'arith_type1'), 'Boolean'),
      | set_param(gcb, 'bitwidth1', '1');
      | set_param(gcb, 'bin_pt1', '0');
      | a(6) = {'off'};
      | a(8) = {'off'};
      | else
      | a(6) = {'on'};
      | a(8) = {'on'};
      | end
      | set_param(gcb, 'MaskEnables', a);
      | |
      mask TabNameString: Setup,Setup,Setup,Setup,IO1,IO1,IO1,IO1
  count : Subsystem  Tag=xps:sw_reg  AncestorBlock=xps_library/software register  mask{numios=1, io_dir=To Processor, io_delay=0, sample_period=1, name1=reg, bitwidth1=32, arith_type1=Unsigned, bin_pt1=0}
      mask prompts: numios "Num I/Os"; io_dir "I/O direction"; io_delay "I/O delay"; sample_period "Sample period"; name1 "Name"; bitwidth1 "Bitwidth"; arith_type1 "Type"; bin_pt1 "Binary pt"
      mask init:
      | swreg_init(gcb);
      mask StyleString: edit,popup(To Processor|From Processor),edit,edit,edit,edit,popup(Unsigned|Signed  (2's comp)|Boolean),edit
      mask CallbackString:
      | t = timer('StartDelay', 0.1); t.TimerFcn = {@swreg_cb, gcb}; start(t);|a = get_param(gcb, 'MaskEnables');
      | if strcmp(get_param(gcb, 'io_dir'), 'To Processor'),
      | a(4) = {'off'};
      | else
      | a(4) = {'on'};
      | end
      | set_param(gcb, 'MaskEnables', a);
      | |||||a = get_param(gcb, 'MaskEnables');
      | if strcmp(get_param(gcb, 'arith_type1'), 'Boolean'),
      | set_param(gcb, 'bitwidth1', '1');
      | set_param(gcb, 'bin_pt1', '0');
      | a(6) = {'off'};
      | a(8) = {'off'};
      | else
      | a(6) = {'on'};
      | a(8) = {'on'};
      | end
      | set_param(gcb, 'MaskEnables', a);
      | |
      mask TabNameString: Setup,Setup,Setup,Setup,IO1,IO1,IO1,IO1
  div_200e6 : xbsIndex_r4/Counter  cnt_type=Count Limited  cnt_to=200e6-1  operation=Up  start_count=0  cnt_by_val=1  arith_type=Unsigned  n_bits=nextpow2(200e6)  bin_pt=0  load_pin=off  rst=off  en=off  explicit_period=on  period=1  dbl_ovrd=off  use_behavioral_HDL=on  implementation=Fabric  block_type=counter
  edge_detect : Subsystem  AncestorBlock=casper_library_misc/edge_detect  mask(edge_detect){edge=Rising, polarity=Active High, x_in=[0 0.08 0.08 0.16 0.16 0.24 0.24 0.32 0.32 0.4], y_in=[-1 -1  1  1  1  1  1  1  1  1], x_out=[0.6 0.68 0.68 0.76 0.76 0.84 0.84 0.92 0.92 1], y_out=[-1 -1  1  1 -1 -1 -1 -1 -1 -1]}
      mask prompts: edge "Edge Type"; polarity "Output Polarity"; x_in "Input X Positions"; y_in "Input Y Positions"; x_out "Output X Positions"; y_out "Output Y Positions"
      mask help: Output is active for one-cycle after each edge of the specified type (i.e. rising, falling or both).
      mask init:
      | edge_detect_init(gcb,...
      |     'edge',edge,...
      |     'polarity',polarity,...
      |     'x_in',x_in,...
      |     'x_out',x_out,...
      |     'y_in',y_in,...
      |     'y_out',y_out);
      | 
      | x_in_num=str2num(getfield(getfield(get_param(gcb,'UserData'),'parameters'),'x_in'));
      | x_out_num=str2num(getfield(getfield(get_param(gcb,'UserData'),'parameters'),'x_out'));
      | y_in_num=str2num(getfield(getfield(get_param(gcb,'UserData'),'parameters'),'y_in'));
      | y_out_num=str2num(getfield(getfield(get_param(gcb,'UserData'),'parameters'),'y_out'));
      settings: AttributesFormatString=|
      | rising edges
      | active high
      mask Help: Output is active for one-cycle after each edge of the specified type (i.e. rising, falling, or both).  Latency is zero.
      mask StyleString: popup(Rising|Falling|Both),popup(Active High|Active Low),edit,edit,edit,edit
      mask Display:
      | plot(x_in_num,0.5+y_in_num*0.2);
      | plot(x_out_num,0.5+y_out_num*0.2);
  edge_detect1 : Subsystem  AncestorBlock=casper_library_misc/edge_detect  mask(edge_detect){edge=Falling, polarity=Active High, x_in=[0 0.08 0.08 0.16 0.16 0.24 0.24 0.32 0.32 0.4], y_in=[-1 -1  1  1  1  1  1  1  1  1], x_out=[0.6 0.68 0.68 0.76 0.76 0.84 0.84 0.92 0.92 1], y_out=[-1 -1  1  1 -1 -1 -1 -1 -1 -1]}
      mask prompts: edge "Edge Type"; polarity "Output Polarity"; x_in "Input X Positions"; y_in "Input Y Positions"; x_out "Output X Positions"; y_out "Output Y Positions"
      mask help: Output is active for one-cycle after each edge of the specified type (i.e. rising, falling or both).
      mask init: same as roach2_sin/sync/edge_detect
      settings: AttributesFormatString=|
      | falling edges
      | active high
      mask Help: Output is active for one-cycle after each edge of the specified type (i.e. rising, falling, or both).  Latency is zero.
      mask StyleString: popup(Rising|Falling|Both),popup(Active High|Active Low),edit,edit,edit,edit
      mask Display:
      | plot(x_in_num,0.5+y_in_num*0.2);
      | plot(x_out_num,0.5+y_out_num*0.2);
  msb : xbsIndex_r4/Slice  nbits=1  boolean_output=on  mode=Upper Bit Location + Width  bit1=0  base1=MSB of Input  bit0=0  base0=LSB of Input  dbl_ovrd=off  block_type=slice
  period : Subsystem  Tag=xps:sw_reg  AncestorBlock=xps_library/software register  mask{numios=1, io_dir=To Processor, io_delay=0, sample_period=1, name1=reg, bitwidth1=32, arith_type1=Unsigned, bin_pt1=0}
      mask prompts: numios "Num I/Os"; io_dir "I/O direction"; io_delay "I/O delay"; sample_period "Sample period"; name1 "Name"; bitwidth1 "Bitwidth"; arith_type1 "Type"; bin_pt1 "Binary pt"
      mask init:
      | swreg_init(gcb);
      mask StyleString: edit,popup(To Processor|From Processor),edit,edit,edit,edit,popup(Unsigned|Signed  (2's comp)|Boolean),edit
      mask CallbackString:
      | t = timer('StartDelay', 0.1); t.TimerFcn = {@swreg_cb, gcb}; start(t);|a = get_param(gcb, 'MaskEnables');
      | if strcmp(get_param(gcb, 'io_dir'), 'To Processor'),
      | a(4) = {'off'};
      | else
      | a(4) = {'on'};
      | end
      | set_param(gcb, 'MaskEnables', a);
      | |||||a = get_param(gcb, 'MaskEnables');
      | if strcmp(get_param(gcb, 'arith_type1'), 'Boolean'),
      | set_param(gcb, 'bitwidth1', '1');
      | set_param(gcb, 'bin_pt1', '0');
      | a(6) = {'off'};
      | a(8) = {'off'};
      | else
      | a(6) = {'on'};
      | a(8) = {'on'};
      | end
      | set_param(gcb, 'MaskEnables', a);
      | |
      mask TabNameString: Setup,Setup,Setup,Setup,IO1,IO1,IO1,IO1
  sync_count : xbsIndex_r4/Counter  cnt_type=Free Running  cnt_to=Inf  operation=Up  start_count=0  cnt_by_val=1  arith_type=Unsigned  n_bits=32  bin_pt=0  load_pin=off  rst=off  en=on  explicit_period=on  period=1  dbl_ovrd=off  use_behavioral_HDL=on  implementation=Fabric  block_type=counter
  sync_in : xps_library/gpio  Tag=xps:gpio  io_group=ROACH2:sync_in  io_dir=in  arith_type=Boolean  bitwidth=1  bin_pt=0  bit_index=0  sample_period=1  use_single_ended=off  use_ddr=off  reg_iob=on  reg_clk_phase=0  termination=None
  sync_period : xbsIndex_r4/Counter  cnt_type=Free Running  cnt_to=Inf  operation=Up  start_count=1  cnt_by_val=1  arith_type=Unsigned  n_bits=32  bin_pt=0  load_pin=off  rst=on  en=off  explicit_period=on  period=1  dbl_ovrd=off  use_behavioral_HDL=on  implementation=Fabric  block_type=counter
  uptime : Subsystem  Tag=xps:sw_reg  AncestorBlock=xps_library/software register  mask{numios=1, io_dir=To Processor, io_delay=0, sample_period=1, name1=reg, bitwidth1=32, arith_type1=Unsigned, bin_pt1=0}
      mask prompts: numios "Num I/Os"; io_dir "I/O direction"; io_delay "I/O delay"; sample_period "Sample period"; name1 "Name"; bitwidth1 "Bitwidth"; arith_type1 "Type"; bin_pt1 "Binary pt"
      mask init:
      | swreg_init(gcb);
      mask StyleString: edit,popup(To Processor|From Processor),edit,edit,edit,edit,popup(Unsigned|Signed  (2's comp)|Boolean),edit
      mask CallbackString:
      | t = timer('StartDelay', 0.1); t.TimerFcn = {@swreg_cb, gcb}; start(t);|a = get_param(gcb, 'MaskEnables');
      | if strcmp(get_param(gcb, 'io_dir'), 'To Processor'),
      | a(4) = {'off'};
      | else
      | a(4) = {'on'};
      | end
      | set_param(gcb, 'MaskEnables', a);
      | |||||a = get_param(gcb, 'MaskEnables');
      | if strcmp(get_param(gcb, 'arith_type1'), 'Boolean'),
      | set_param(gcb, 'bitwidth1', '1');
      | set_param(gcb, 'bin_pt1', '0');
      | a(6) = {'off'};
      | a(8) = {'off'};
      | else
      | a(6) = {'on'};
      | a(8) = {'on'};
      | end
      | set_param(gcb, 'MaskEnables', a);
      | |
      mask TabNameString: Setup,Setup,Setup,Setup,IO1,IO1,IO1,IO1
  uptime_count : xbsIndex_r4/Counter  cnt_type=Free Running  cnt_to=Inf  operation=Up  start_count=0  cnt_by_val=1  arith_type=Unsigned  n_bits=32  bin_pt=0  load_pin=off  rst=off  en=on  explicit_period=on  period=1  dbl_ovrd=off  use_behavioral_HDL=on  implementation=Fabric  block_type=counter
  tag scope: arm_noise=global, arm_sync=global, sw_sync=global, sync_gpio_in=global
  wires:
  sync_in/1 -> Register/1, Register3/1
  Register/1 -> [sync_gpio_in]
  sync_period/1 -> Register1/1
  sync_count/1 -> Register2/1
  Register1/1 -> period/1(out_reg)
  count/1(sim_out) -> Terminator/1
  period/1(sim_out) -> Terminator1/1
  Register2/1 -> count/1(out_reg)
  edge_detect/1(out) -> Register1/2, sync_period/1, sync_count/1
  Constant33/1 -> sync_in/1
  Constant/1 -> arm/1(sim_reg)
  BitBasher/1 -> Convert1/1
  BitBasher/2 -> Convert2/1
  BitBasher/3 -> Convert3/1
  Convert1/1 -> [sw_sync]
  Convert2/1 -> [arm_noise]
  Convert3/1 -> [arm_sync]
  arm/1(in_reg) -> BitBasher/1
  Register3/1 -> edge_detect/1(in)
  uptime/1(sim_out) -> Terminator2/1
  Register4/1 -> uptime/1(out_reg)
  uptime_count/1 -> Register4/1
  edge_detect1/1(out) -> uptime_count/1
  div_200e6/1 -> msb/1
  msb/1 -> edge_detect1/1(in)

== system roach2_sin/timebase ==
  note: This circuit implements a scheme for synchronizing
      | multiple FPGAs to within 1 clock cycle.  "arm_rst"
      | comes from the "feng_ctrl" register, and indicates that
      | the next rising edge of "sync_rst" will generate an
      | internal sync pulse.
      |
      | "sync_rst" may be generated from the "feng_ctrl"
      | register, but more often, a 1 PPS signal is supplied to
      | the "sync_in" input of the ROACH2 for this purpose.
      |
      | Once a sync pulse is generated, "sync_gen" generates a
      | single pulse every 2^27 clocks that is used only to
      | provide visual confirmation of the synchronization.
      |
      | LED2 provides visual status of the synchronization.
      | While armed, LED2 will be on.  Once a sync pulse
      | arrives, LED2 will blink once every 2^28 clock cycles
      | (1.34217728 seconds).
  Logical7 : xbsIndex_r4/Logical  logical_function=OR  inputs=2  en=off  latency=1  precision=Full  arith_type=Unsigned  n_bits=8  bin_pt=2  align_bp=on  dbl_ovrd=off  block_type=logical
      settings: xl_area=[0, 0, 0, 0, 0, 0, 0]
  sync_gen : Subsystem
  tag scope: sync_gen=global, sync_in=global
  wires:
  [sync_gpio_in] -> Logical7/1
  Logical7/1 -> sync_gen/1(sync_in), [sync_in]
  sync_gen/1(sync_out) -> [sync_gen]
  [sw_sync] -> Logical7/2
  [arm_sync] -> sync_gen/2(arm)
